simulink model "wlan_phy_rx_pmd"  (Simulink 7.8) (continued)
== system wlan_phy_rx_pmd/EDK Processor == (continued)
      | signal fifo_bank_out_i      : std_logic_vector(C_S_AXI_DATA_WIDTH-1 downto 0);
      | signal shmem_bank_out_i     : std_logic_vector(C_S_AXI_DATA_WIDTH-1 downto 0);
      |     
      | -- 'From Register'
      | -- 'RX_PKT_RSSI_CD'
      | signal sm_RX_PKT_RSSI_CD_dout_i  : std_logic_vector(C_S_AXI_DATA_WIDTH-1 downto 0);
      | -- 'RX_PKT_AGC_GAINS'
      | signal sm_RX_PKT_AGC_GAINS_dout_i  : std_logic_vector(C_S_AXI_DATA_WIDTH-1 downto 0);
      | -- 'RX_PKT_RSSI_AB'
      | signal sm_RX_PKT_RSSI_AB_dout_i  : std_logic_vector(C_S_AXI_DATA_WIDTH-1 downto 0);
      | -- 'CFO_EST_TIME_DOMAIN'
      | signal sm_CFO_EST_TIME_DOMAIN_dout_i  : std_logic_vector(C_S_AXI_DATA_WIDTH-1 downto 0);
      | -- 'Status'
      | signal sm_Status_dout_i  : std_logic_vector(C_S_AXI_DATA_WIDTH-1 downto 0);
      | -- 'To Register'
      | -- 'DEBUG_GPIO'
      | signal sm_DEBUG_GPIO_din_i   : std_logic_vector(C_S_AXI_DATA_WIDTH-1 downto 0);
      | signal sm_DEBUG_GPIO_en_i    : std_logic;
      | signal sm_DEBUG_GPIO_dout_i  : std_logic_vector(C_S_AXI_DATA_WIDTH-1 downto 0);
      | -- 'RSSI_THRESH'
      | signal sm_RSSI_THRESH_din_i   : std_logic_vector(C_S_AXI_DATA_WIDTH-1 downto 0);
      | signal sm_RSSI_THRESH_en_i    : std_logic;
      | signal sm_RSSI_THRESH_dout_i  : std_logic_vector(C_S_AXI_DATA_WIDTH-1 downto 0);
      | -- 'CONFIG'
      | signal sm_CONFIG_din_i   : std_logic_vector(C_S_AXI_DATA_WIDTH-1 downto 0);
      | signal sm_CONFIG_en_i    : std_logic;
      | signal sm_CONFIG_dout_i  : std_logic_vector(C_S_AXI_DATA_WIDTH-1 downto 0);
      | -- 'DSSS_RX_CONFIG'
      | signal sm_DSSS_RX_CONFIG_din_i   : std_logic_vector(C_S_AXI_DATA_WIDTH-1 downto 0);
      | signal sm_DSSS_RX_CONFIG_en_i    : std_logic;
      | signal sm_DSSS_RX_CONFIG_dout_i  : std_logic_vector(C_S_AXI_DATA_WIDTH-1 downto 0);
      | -- 'PKT_BUF_SEL'
      | signal sm_PKT_BUF_SEL_din_i   : std_logic_vector(C_S_AXI_DATA_WIDTH-1 downto 0);
      | signal sm_PKT_BUF_SEL_en_i    : std_logic;
      | signal sm_PKT_BUF_SEL_dout_i  : std_logic_vector(C_S_AXI_DATA_WIDTH-1 downto 0);
      | -- 'PKTDET_AUTOCORR_CONFIG'
      | signal sm_PKTDET_AUTOCORR_CONFIG_din_i   : std_logic_vector(C_S_AXI_DATA_WIDTH-1 downto 0);
      | signal sm_PKTDET_AUTOCORR_CONFIG_en_i    : std_logic;
      | signal sm_PKTDET_AUTOCORR_CONFIG_dout_i  : std_logic_vector(C_S_AXI_DATA_WIDTH-1 downto 0);
      | -- 'FEC_CONFIG'
      | signal sm_FEC_CONFIG_din_i   : std_logic_vector(C_S_AXI_DATA_WIDTH-1 downto 0);
      | signal sm_FEC_CONFIG_en_i    : std_logic;
      | signal sm_FEC_CONFIG_dout_i  : std_logic_vector(C_S_AXI_DATA_WIDTH-1 downto 0);
      | -- 'Control'
      | signal sm_Control_din_i   : std_logic_vector(C_S_AXI_DATA_WIDTH-1 downto 0);
      | signal sm_Control_en_i    : std_logic;
      | signal sm_Control_dout_i  : std_logic_vector(C_S_AXI_DATA_WIDTH-1 downto 0);
      | -- 'CHAN_EST_SMOOTHING'
      | signal sm_CHAN_EST_SMOOTHING_din_i   : std_logic_vector(C_S_AXI_DATA_WIDTH-1 downto 0);
      | signal sm_CHAN_EST_SMOOTHING_en_i    : std_logic;
      | signal sm_CHAN_EST_SMOOTHING_dout_i  : std_logic_vector(C_S_AXI_DATA_WIDTH-1 downto 0);
      | -- 'LTS_Corr_Thresh'
      | signal sm_LTS_Corr_Thresh_din_i   : std_logic_vector(C_S_AXI_DATA_WIDTH-1 downto 0);
      | signal sm_LTS_Corr_Thresh_en_i    : std_logic;
      | signal sm_LTS_Corr_Thresh_dout_i  : std_logic_vector(C_S_AXI_DATA_WIDTH-1 downto 0);
      | -- 'PKTDET_DSSS_CONFIG'
      | signal sm_PKTDET_DSSS_CONFIG_din_i   : std_logic_vector(C_S_AXI_DATA_WIDTH-1 downto 0);
      | signal sm_PKTDET_DSSS_CONFIG_en_i    : std_logic;
      | signal sm_PKTDET_DSSS_CONFIG_dout_i  : std_logic_vector(C_S_AXI_DATA_WIDTH-1 downto 0);
      | -- 'LTS_Corr_Config'
      | signal sm_LTS_Corr_Config_din_i   : std_logic_vector(C_S_AXI_DATA_WIDTH-1 downto 0);
      | signal sm_LTS_Corr_Config_en_i    : std_logic;
      | signal sm_LTS_Corr_Config_dout_i  : std_logic_vector(C_S_AXI_DATA_WIDTH-1 downto 0);
      | -- 'PHY_CCA_CONFIG'
      | signal sm_PHY_CCA_CONFIG_din_i   : std_logic_vector(C_S_AXI_DATA_WIDTH-1 downto 0);
      | signal sm_PHY_CCA_CONFIG_en_i    : std_logic;
      | signal sm_PHY_CCA_CONFIG_dout_i  : std_logic_vector(C_S_AXI_DATA_WIDTH-1 downto 0);
      | -- 'PKTBUF_MAX_WRITE_ADDR'
      | signal sm_PKTBUF_MAX_WRITE_ADDR_din_i   : std_logic_vector(C_S_AXI_DATA_WIDTH-1 downto 0);
      | signal sm_PKTBUF_MAX_WRITE_ADDR_en_i    : std_logic;
      | signal sm_PKTBUF_MAX_WRITE_ADDR_dout_i  : std_logic_vector(C_S_AXI_DATA_WIDTH-1 downto 0);
      | -- 'FFT_Config'
      | signal sm_FFT_Config_din_i   : std_logic_vector(C_S_AXI_DATA_WIDTH-1 downto 0);
      | signal sm_FFT_Config_en_i    : std_logic;
      | signal sm_FFT_Config_dout_i  : std_logic_vector(C_S_AXI_DATA_WIDTH-1 downto 0);
      | -- 'PKTDET_RSSI_CONFIG'
      | signal sm_PKTDET_RSSI_CONFIG_din_i   : std_logic_vector(C_S_AXI_DATA_WIDTH-1 downto 0);
      | signal sm_PKTDET_RSSI_CONFIG_en_i    : std_logic;
      | signal sm_PKTDET_RSSI_CONFIG_dout_i  : std_logic_vector(C_S_AXI_DATA_WIDTH-1 downto 0);
      | -- 'From FIFO'
      | -- 'To FIFO'
      | -- 'Shared Memory'
      | 
      | type t_read_state is (IDLE, READ_PREP, READ_DATA);
      | signal read_state : t_read_state;
      | 
      | type t_write_state is (IDLE, WRITE_DATA, WRITE_RESPONSE);
      | signal write_state : t_write_state;
      | 
      | type t_memmap_state is (READ, WRITE);
      | signal memmap_state : t_memmap_state;
      | 
      | constant C_READ_PREP_DELAY : std_logic_vector(1 downto 0) := "11";
      | 
      | signal read_prep_counter : std_logic_vector(1 downto 0);
      | signal read_addr_counter : std_logic_vector(C_S_AXI_ARLEN_WIDTH-1 downto 0);
      | signal read_data_counter : std_logic_vector(C_S_AXI_ARLEN_WIDTH-1 downto 0);
      | 
      | -- enable of shared BRAMs
      | signal s_shram_en : std_logic;
      | 
      | signal write_addr_valid : std_logic;
      | signal write_ready : std_logic;
      | 
      | -- 're' of From/To FIFOs
      | signal s_fifo_re : std_logic;
      | -- 'we' of To FIFOs
      | signal s_fifo_we : std_logic;
      | 
      | begin
      | 
      | -- enable for 'Shared Memory' blocks
      | 
      | -- conversion to match with the data bus width
      | -- 'From Register'
      | -- 'RX_PKT_RSSI_CD'
      | gen_sm_RX_PKT_RSSI_CD_dout_i: if (32 < C_S_AXI_DATA_WIDTH) generate
      |     sm_RX_PKT_RSSI_CD_dout_i(C_S_AXI_DATA_WIDTH-1 downto 32) <= (others => '0');
      | end generate gen_sm_RX_PKT_RSSI_CD_dout_i;
      | sm_RX_PKT_RSSI_CD_dout_i(32-1 downto 0) <= sm_RX_PKT_RSSI_CD_dout;
      | -- 'RX_PKT_AGC_GAINS'
      | gen_sm_RX_PKT_AGC_GAINS_dout_i: if (32 < C_S_AXI_DATA_WIDTH) generate
      |     sm_RX_PKT_AGC_GAINS_dout_i(C_S_AXI_DATA_WIDTH-1 downto 32) <= (others => '0');
      | end generate gen_sm_RX_PKT_AGC_GAINS_dout_i;
      | sm_RX_PKT_AGC_GAINS_dout_i(32-1 downto 0) <= sm_RX_PKT_AGC_GAINS_dout;
      | -- 'RX_PKT_RSSI_AB'
      | gen_sm_RX_PKT_RSSI_AB_dout_i: if (32 < C_S_AXI_DATA_WIDTH) generate
      |     sm_RX_PKT_RSSI_AB_dout_i(C_S_AXI_DATA_WIDTH-1 downto 32) <= (others => '0');
      | end generate gen_sm_RX_PKT_RSSI_AB_dout_i;
      | sm_RX_PKT_RSSI_AB_dout_i(32-1 downto 0) <= sm_RX_PKT_RSSI_AB_dout;
      | -- 'CFO_EST_TIME_DOMAIN'
      | gen_sm_CFO_EST_TIME_DOMAIN_dout_i: if (32 < C_S_AXI_DATA_WIDTH) generate
      |     sm_CFO_EST_TIME_DOMAIN_dout_i(C_S_AXI_DATA_WIDTH-1 downto 32) <= (others => '0');
      | end generate gen_sm_CFO_EST_TIME_DOMAIN_dout_i;
      | sm_CFO_EST_TIME_DOMAIN_dout_i(32-1 downto 0) <= sm_CFO_EST_TIME_DOMAIN_dout;
      | -- 'Status'
      | gen_sm_Status_dout_i: if (32 < C_S_AXI_DATA_WIDTH) generate
      |     sm_Status_dout_i(C_S_AXI_DATA_WIDTH-1 downto 32) <= (others => '0');
      | end generate gen_sm_Status_dout_i;
      | sm_Status_dout_i(32-1 downto 0) <= sm_Status_dout;
      | -- 'To Register'
      | -- 'DEBUG_GPIO'
      | sm_DEBUG_GPIO_din     <= sm_DEBUG_GPIO_din_i(32-1 downto 0);
      | sm_DEBUG_GPIO_en      <= sm_DEBUG_GPIO_en_i;
      | gen_sm_DEBUG_GPIO_dout_i: if (32 < C_S_AXI_DATA_WIDTH) generate
      |     sm_DEBUG_GPIO_dout_i(C_S_AXI_DATA_WIDTH-1 downto 32) <= (others => '0');
      | end generate gen_sm_DEBUG_GPIO_dout_i;
      | sm_DEBUG_GPIO_dout_i(32-1 downto 0) <= sm_DEBUG_GPIO_dout;
      | -- 'RSSI_THRESH'
      | sm_RSSI_THRESH_din     <= sm_RSSI_THRESH_din_i(32-1 downto 0);
      | sm_RSSI_THRESH_en      <= sm_RSSI_THRESH_en_i;
      | gen_sm_RSSI_THRESH_dout_i: if (32 < C_S_AXI_DATA_WIDTH) generate
      |     sm_RSSI_THRESH_dout_i(C_S_AXI_DATA_WIDTH-1 downto 32) <= (others => '0');
      | end generate gen_sm_RSSI_THRESH_dout_i;
      | sm_RSSI_THRESH_dout_i(32-1 downto 0) <= sm_RSSI_THRESH_dout;
      | -- 'CONFIG'
      | sm_CONFIG_din     <= sm_CONFIG_din_i(32-1 downto 0);
      | sm_CONFIG_en      <= sm_CONFIG_en_i;
      | gen_sm_CONFIG_dout_i: if (32 < C_S_AXI_DATA_WIDTH) generate
      |     sm_CONFIG_dout_i(C_S_AXI_DATA_WIDTH-1 downto 32) <= (others => '0');
      | end generate gen_sm_CONFIG_dout_i;
      | sm_CONFIG_dout_i(32-1 downto 0) <= sm_CONFIG_dout;
      | -- 'DSSS_RX_CONFIG'
      | sm_DSSS_RX_CONFIG_din     <= sm_DSSS_RX_CONFIG_din_i(32-1 downto 0);
      | sm_DSSS_RX_CONFIG_en      <= sm_DSSS_RX_CONFIG_en_i;
      | gen_sm_DSSS_RX_CONFIG_dout_i: if (32 < C_S_AXI_DATA_WIDTH) generate
      |     sm_DSSS_RX_CONFIG_dout_i(C_S_AXI_DATA_WIDTH-1 downto 32) <= (others => '0');
      | end generate gen_sm_DSSS_RX_CONFIG_dout_i;
      | sm_DSSS_RX_CONFIG_dout_i(32-1 downto 0) <= sm_DSSS_RX_CONFIG_dout;
      | -- 'PKT_BUF_SEL'
      | sm_PKT_BUF_SEL_din     <= sm_PKT_BUF_SEL_din_i(32-1 downto 0);
      | sm_PKT_BUF_SEL_en      <= sm_PKT_BUF_SEL_en_i;
      | gen_sm_PKT_BUF_SEL_dout_i: if (32 < C_S_AXI_DATA_WIDTH) generate
      |     sm_PKT_BUF_SEL_dout_i(C_S_AXI_DATA_WIDTH-1 downto 32) <= (others => '0');
      | end generate gen_sm_PKT_BUF_SEL_dout_i;
      | sm_PKT_BUF_SEL_dout_i(32-1 downto 0) <= sm_PKT_BUF_SEL_dout;
      | -- 'PKTDET_AUTOCORR_CONFIG'
      | sm_PKTDET_AUTOCORR_CONFIG_din     <= sm_PKTDET_AUTOCORR_CONFIG_din_i(32-1 downto 0);
      | sm_PKTDET_AUTOCORR_CONFIG_en      <= sm_PKTDET_AUTOCORR_CONFIG_en_i;
      | gen_sm_PKTDET_AUTOCORR_CONFIG_dout_i: if (32 < C_S_AXI_DATA_WIDTH) generate
      |     sm_PKTDET_AUTOCORR_CONFIG_dout_i(C_S_AXI_DATA_WIDTH-1 downto 32) <= (others => '0');
      | end generate gen_sm_PKTDET_AUTOCORR_CONFIG_dout_i;
      | sm_PKTDET_AUTOCORR_CONFIG_dout_i(32-1 downto 0) <= sm_PKTDET_AUTOCORR_CONFIG_dout;
      | -- 'FEC_CONFIG'
      | sm_FEC_CONFIG_din     <= sm_FEC_CONFIG_din_i(32-1 downto 0);
      | sm_FEC_CONFIG_en      <= sm_FEC_CONFIG_en_i;
      | gen_sm_FEC_CONFIG_dout_i: if (32 < C_S_AXI_DATA_WIDTH) generate
      |     sm_FEC_CONFIG_dout_i(C_S_AXI_DATA_WIDTH-1 downto 32) <= (others => '0');
      | end generate gen_sm_FEC_CONFIG_dout_i;
      | sm_FEC_CONFIG_dout_i(32-1 downto 0) <= sm_FEC_CONFIG_dout;
      | -- 'Control'
      | sm_Control_din     <= sm_Control_din_i(32-1 downto 0);
      | sm_Control_en      <= sm_Control_en_i;
      | gen_sm_Control_dout_i: if (32 < C_S_AXI_DATA_WIDTH) generate
      |     sm_Control_dout_i(C_S_AXI_DATA_WIDTH-1 downto 32) <= (others => '0');
      | end generate gen_sm_Control_dout_i;
      | sm_Control_dout_i(32-1 downto 0) <= sm_Control_dout;
      | -- 'CHAN_EST_SMOOTHING'
      | sm_CHAN_EST_SMOOTHING_din     <= sm_CHAN_EST_SMOOTHING_din_i(32-1 downto 0);
      | sm_CHAN_EST_SMOOTHING_en      <= sm_CHAN_EST_SMOOTHING_en_i;
      | gen_sm_CHAN_EST_SMOOTHING_dout_i: if (32 < C_S_AXI_DATA_WIDTH) generate
      |     sm_CHAN_EST_SMOOTHING_dout_i(C_S_AXI_DATA_WIDTH-1 downto 32) <= (others => '0');
      | end generate gen_sm_CHAN_EST_SMOOTHING_dout_i;
      | sm_CHAN_EST_SMOOTHING_dout_i(32-1 downto 0) <= sm_CHAN_EST_SMOOTHING_dout;
      | -- 'LTS_Corr_Thresh'
      | sm_LTS_Corr_Thresh_din     <= sm_LTS_Corr_Thresh_din_i(32-1 downto 0);
      | sm_LTS_Corr_Thresh_en      <= sm_LTS_Corr_Thresh_en_i;
      | gen_sm_LTS_Corr_Thresh_dout_i: if (32 < C_S_AXI_DATA_WIDTH) generate
      |     sm_LTS_Corr_Thresh_dout_i(C_S_AXI_DATA_WIDTH-1 downto 32) <= (others => '0');
      | end generate gen_sm_LTS_Corr_Thresh_dout_i;
      | sm_LTS_Corr_Thresh_dout_i(32-1 downto 0) <= sm_LTS_Corr_Thresh_dout;
      | -- 'PKTDET_DSSS_CONFIG'
      | sm_PKTDET_DSSS_CONFIG_din     <= sm_PKTDET_DSSS_CONFIG_din_i(32-1 downto 0);
      | sm_PKTDET_DSSS_CONFIG_en      <= sm_PKTDET_DSSS_CONFIG_en_i;
      | gen_sm_PKTDET_DSSS_CONFIG_dout_i: if (32 < C_S_AXI_DATA_WIDTH) generate
      |     sm_PKTDET_DSSS_CONFIG_dout_i(C_S_AXI_DATA_WIDTH-1 downto 32) <= (others => '0');
      | end generate gen_sm_PKTDET_DSSS_CONFIG_dout_i;
      | sm_PKTDET_DSSS_CONFIG_dout_i(32-1 downto 0) <= sm_PKTDET_DSSS_CONFIG_dout;
      | -- 'LTS_Corr_Config'
      | sm_LTS_Corr_Config_din     <= sm_LTS_Corr_Config_din_i(32-1 downto 0);
      | sm_LTS_Corr_Config_en      <= sm_LTS_Corr_Config_en_i;
      | gen_sm_LTS_Corr_Config_dout_i: if (32 < C_S_AXI_DATA_WIDTH) generate
      |     sm_LTS_Corr_Config_dout_i(C_S_AXI_DATA_WIDTH-1 downto 32) <= (others => '0');
      | end generate gen_sm_LTS_Corr_Config_dout_i;
      | sm_LTS_Corr_Config_dout_i(32-1 downto 0) <= sm_LTS_Corr_Config_dout;
      | -- 'PHY_CCA_CONFIG'
      | sm_PHY_CCA_CONFIG_din     <= sm_PHY_CCA_CONFIG_din_i(32-1 downto 0);
      | sm_PHY_CCA_CONFIG_en      <= sm_PHY_CCA_CONFIG_en_i;
      | gen_sm_PHY_CCA_CONFIG_dout_i: if (32 < C_S_AXI_DATA_WIDTH) generate
      |     sm_PHY_CCA_CONFIG_dout_i(C_S_AXI_DATA_WIDTH-1 downto 32) <= (others => '0');
      | end generate gen_sm_PHY_CCA_CONFIG_dout_i;
      | sm_PHY_CCA_CONFIG_dout_i(32-1 downto 0) <= sm_PHY_CCA_CONFIG_dout;
      | -- 'PKTBUF_MAX_WRITE_ADDR'
      | sm_PKTBUF_MAX_WRITE_ADDR_din     <= sm_PKTBUF_MAX_WRITE_ADDR_din_i(32-1 downto 0);
      | sm_PKTBUF_MAX_WRITE_ADDR_en      <= sm_PKTBUF_MAX_WRITE_ADDR_en_i;
      | gen_sm_PKTBUF_MAX_WRITE_ADDR_dout_i: if (32 < C_S_AXI_DATA_WIDTH) generate
      |     sm_PKTBUF_MAX_WRITE_ADDR_dout_i(C_S_AXI_DATA_WIDTH-1 downto 32) <= (others => '0');
      | end generate gen_sm_PKTBUF_MAX_WRITE_ADDR_dout_i;
      | sm_PKTBUF_MAX_WRITE_ADDR_dout_i(32-1 downto 0) <= sm_PKTBUF_MAX_WRITE_ADDR_dout;
      | -- 'FFT_Config'
      | sm_FFT_Config_din     <= sm_FFT_Config_din_i(32-1 downto 0);
      | sm_FFT_Config_en      <= sm_FFT_Config_en_i;
      | gen_sm_FFT_Config_dout_i: if (32 < C_S_AXI_DATA_WIDTH) generate
      |     sm_FFT_Config_dout_i(C_S_AXI_DATA_WIDTH-1 downto 32) <= (others => '0');
      | end generate gen_sm_FFT_Config_dout_i;
      | sm_FFT_Config_dout_i(32-1 downto 0) <= sm_FFT_Config_dout;
      | -- 'PKTDET_RSSI_CONFIG'
      | sm_PKTDET_RSSI_CONFIG_din     <= sm_PKTDET_RSSI_CONFIG_din_i(32-1 downto 0);
      | sm_PKTDET_RSSI_CONFIG_en      <= sm_PKTDET_RSSI_CONFIG_en_i;
      | gen_sm_PKTDET_RSSI_CONFIG_dout_i: if (32 < C_S_AXI_DATA_WIDTH) generate
      |     sm_PKTDET_RSSI_CONFIG_dout_i(C_S_AXI_DATA_WIDTH-1 downto 32) <= (others => '0');
      | end generate gen_sm_PKTDET_RSSI_CONFIG_dout_i;
      | sm_PKTDET_RSSI_CONFIG_dout_i(32-1 downto 0) <= sm_PKTDET_RSSI_CONFIG_dout;
      | -- 'From FIFO'
      | -- 'To FIFO'
      | -- 'Shared Memory'
      | 
      | ReadWriteSelect: process(memmap_state) is begin
      |     if (memmap_state = READ) then
      |     else
      |     end if;
      | end process ReadWriteSelect;
      | 
      | -----------------------------------------------------------------------------
      | -- address for 'Shared Memory'
      | -----------------------------------------------------------------------------
      | SharedMemory_Addr_ResetN : process(AXI_AClk) is begin
      |     if (AXI_AClk'event and AXI_AClk = '1') then
      |         if (AXI_AResetN = '0') then
      |             memmap_state <= READ;
      |         else
      |             if (S_AXI_AWVALID = '1') then
      |                 -- write operation
      |                 memmap_state <= WRITE;
      |             elsif (S_AXI_ARVALID = '1') then
      |                 -- read operation
      |                 memmap_state <= READ;
      |             end if;
      |         end if;
      |     end if;
      | end process SharedMemory_Addr_ResetN;
      | 
      | -----------------------------------------------------------------------------
      | -- WRITE Command Control
      | -----------------------------------------------------------------------------
      | S_AXI_BID     <= S_AXI_BID_i;
      | S_AXI_BVALID  <= S_AXI_BVALID_i;
      | S_AXI_WREADY  <= S_AXI_WREADY_i;
      | -- No error checking
      | S_AXI_BRESP  <= (others=>'0');
      | 
      | PROC_AWREADY_ACK: process(read_state, write_state, S_AXI_ARVALID, S_AXI_AWVALID) is begin
      |     if (write_state = IDLE and S_AXI_AWVALID = '1' and read_state = IDLE) then
      |         S_AXI_AWREADY <= S_AXI_AWVALID;
      |     else
      |         S_AXI_AWREADY <= '0';
      |     end if;
      | end process PROC_AWREADY_ACK;
      | 
      | Cmd_Decode_Write: process(AXI_AClk) is begin
      |     if (AXI_AClk'event and AXI_AClk = '1') then
      |         if (AXI_AResetN = '0') then
      |             write_addr_valid    <= '0';
      |             write_ready         <= '0';
      |             s_fifo_we           <= '0';
      |             S_AXI_BVALID_i      <= '0';
      |             S_AXI_BID_i         <= (others => '0');
      |             write_bank_addr_i   <= (others => '0');
      |             write_linear_addr_i <= (others => '0');
      |         else
      |             if (write_state = IDLE) then
      |                 if (S_AXI_AWVALID = '1' and read_state = IDLE) then
      |                     -- reflect awid
      |                     S_AXI_BID_i <= S_AXI_AWID;
      | 
      |                     -- latch bank and linear addresses
      |                     write_bank_addr_i   <= S_AXI_AWADDR(C_S_AXI_TOTAL_ADDR_LEN-1 downto C_S_AXI_LINEAR_ADDR_LEN+2);
      |                     write_linear_addr_i <= S_AXI_AWADDR(C_S_AXI_LINEAR_ADDR_LEN+1 downto 2);
      |                     write_addr_valid <= '1';
      |                     s_fifo_we <= '1';
      | 
      |                     -- write state transition
      |                     write_state <= WRITE_DATA;
      |                 end if;
      |             elsif (write_state = WRITE_DATA) then
      |                 write_ready <= '1';
      |                 s_fifo_we <= '0';
      |                 write_addr_valid <= S_AXI_WVALID;
      |                 
      |                 if (S_AXI_WVALID = '1' and write_ready = '1') then
      |                     write_linear_addr_i <= Std_Logic_Vector(unsigned(write_linear_addr_i) + 1);
      |                 end if;
      | 
      |                 if (S_AXI_WLAST = '1' and write_ready = '1') then
      |                     -- start responding through B channel upon the last write data sample
      |                     S_AXI_BVALID_i <= '1';
      |                     -- write data is over
      |                     write_addr_valid <= '0';
      |                     write_ready <= '0';
      |                     -- write state transition
      |                     write_state <= WRITE_RESPONSE;
      |                 end if;
      |             elsif (write_state = WRITE_RESPONSE) then
      | 
      |                 if (S_AXI_BREADY = '1') then
      |                     -- write respond is over
      |                     S_AXI_BVALID_i <= '0';
      |                     S_AXI_BID_i <= (others => '0');
      | 
      |                     -- write state transition
      |                     write_state <= IDLE;
      |                 end if;
      |             end if;
      |         end if;
      |     end if;
      | end process Cmd_Decode_Write;
      | 
      | Write_Linear_Addr_Decode : process(AXI_AClk) is 
      | 
      | begin
      |     if (AXI_AClk'event and AXI_AClk = '1') then
      |         if (AXI_AResetN = '0') then
      |             -- 'To Register'
      |             -- DEBUG_GPIO din/en
      |             sm_DEBUG_GPIO_din_i <= (others => '0');
      |             sm_DEBUG_GPIO_en_i <= '0';
      |             -- RSSI_THRESH din/en
      |             sm_RSSI_THRESH_din_i <= (others => '0');
      |             sm_RSSI_THRESH_en_i <= '0';
      |             -- CONFIG din/en
      |             sm_CONFIG_din_i <= (others => '0');
      |             sm_CONFIG_en_i <= '0';
      |             -- DSSS_RX_CONFIG din/en
      |             sm_DSSS_RX_CONFIG_din_i <= (others => '0');
      |             sm_DSSS_RX_CONFIG_en_i <= '0';
      |             -- PKT_BUF_SEL din/en
      |             sm_PKT_BUF_SEL_din_i <= (others => '0');
      |             sm_PKT_BUF_SEL_en_i <= '0';
      |             -- PKTDET_AUTOCORR_CONFIG din/en
      |             sm_PKTDET_AUTOCORR_CONFIG_din_i <= (others => '0');
      |             sm_PKTDET_AUTOCORR_CONFIG_en_i <= '0';
      |             -- FEC_CONFIG din/en
      |             sm_FEC_CONFIG_din_i <= (others => '0');
      |             sm_FEC_CONFIG_en_i <= '0';
      |             -- Control din/en
      |             sm_Control_din_i <= (others => '0');
      |             sm_Control_en_i <= '0';
      |             -- CHAN_EST_SMOOTHING din/en
      |             sm_CHAN_EST_SMOOTHING_din_i <= (others => '0');
      |             sm_CHAN_EST_SMOOTHING_en_i <= '0';
      |             -- LTS_Corr_Thresh din/en
      |             sm_LTS_Corr_Thresh_din_i <= (others => '0');
      |             sm_LTS_Corr_Thresh_en_i <= '0';
      |             -- PKTDET_DSSS_CONFIG din/en
      |             sm_PKTDET_DSSS_CONFIG_din_i <= (others => '0');
      |             sm_PKTDET_DSSS_CONFIG_en_i <= '0';
      |             -- LTS_Corr_Config din/en
      |             sm_LTS_Corr_Config_din_i <= (others => '0');
      |             sm_LTS_Corr_Config_en_i <= '0';
      |             -- PHY_CCA_CONFIG din/en
      |             sm_PHY_CCA_CONFIG_din_i <= (others => '0');
      |             sm_PHY_CCA_CONFIG_en_i <= '0';
      |             -- PKTBUF_MAX_WRITE_ADDR din/en
      |             sm_PKTBUF_MAX_WRITE_ADDR_din_i <= (others => '0');
      |             sm_PKTBUF_MAX_WRITE_ADDR_en_i <= '0';
      |             -- FFT_Config din/en
      |             sm_FFT_Config_din_i <= (others => '0');
      |             sm_FFT_Config_en_i <= '0';
      |             -- PKTDET_RSSI_CONFIG din/en
      |             sm_PKTDET_RSSI_CONFIG_din_i <= (others => '0');
      |             sm_PKTDET_RSSI_CONFIG_en_i <= '0';
      |             -- 'To FIFO'
      |             -- 'Shared Memory'
      |         else
      |             -- default assignments
      | 
      |             -- 'To Register'
      |             if (unsigned(write_bank_addr_i) = 2) then
      |                 if (unsigned(write_linear_addr_i) = 0) then
      |                     -- DEBUG_GPIO din/en
      |                     sm_DEBUG_GPIO_din_i <= S_AXI_WDATA;
      |                     sm_DEBUG_GPIO_en_i  <= write_addr_valid;
      |                 elsif (unsigned(write_linear_addr_i) = 1) then
      |                     -- RSSI_THRESH din/en
      |                     sm_RSSI_THRESH_din_i <= S_AXI_WDATA;
      |                     sm_RSSI_THRESH_en_i  <= write_addr_valid;
      |                 elsif (unsigned(write_linear_addr_i) = 2) then
      |                     -- CONFIG din/en
      |                     sm_CONFIG_din_i <= S_AXI_WDATA;
      |                     sm_CONFIG_en_i  <= write_addr_valid;
      |                 elsif (unsigned(write_linear_addr_i) = 3) then
      |                     -- DSSS_RX_CONFIG din/en
      |                     sm_DSSS_RX_CONFIG_din_i <= S_AXI_WDATA;
      |                     sm_DSSS_RX_CONFIG_en_i  <= write_addr_valid;
      |                 elsif (unsigned(write_linear_addr_i) = 4) then
      |                     -- PKT_BUF_SEL din/en
      |                     sm_PKT_BUF_SEL_din_i <= S_AXI_WDATA;
      |                     sm_PKT_BUF_SEL_en_i  <= write_addr_valid;
      |                 elsif (unsigned(write_linear_addr_i) = 5) then
      |                     -- PKTDET_AUTOCORR_CONFIG din/en
      |                     sm_PKTDET_AUTOCORR_CONFIG_din_i <= S_AXI_WDATA;
      |                     sm_PKTDET_AUTOCORR_CONFIG_en_i  <= write_addr_valid;
      |                 elsif (unsigned(write_linear_addr_i) = 6) then
      |                     -- FEC_CONFIG din/en
      |                     sm_FEC_CONFIG_din_i <= S_AXI_WDATA;
      |                     sm_FEC_CONFIG_en_i  <= write_addr_valid;
      |                 elsif (unsigned(write_linear_addr_i) = 7) then
      |                     -- Control din/en
      |                     sm_Control_din_i <= S_AXI_WDATA;
      |                     sm_Control_en_i  <= write_addr_valid;
      |                 elsif (unsigned(write_linear_addr_i) = 8) then
      |                     -- CHAN_EST_SMOOTHING din/en
      |                     sm_CHAN_EST_SMOOTHING_din_i <= S_AXI_WDATA;
      |                     sm_CHAN_EST_SMOOTHING_en_i  <= write_addr_valid;
      |                 elsif (unsigned(write_linear_addr_i) = 9) then
      |                     -- LTS_Corr_Thresh din/en
      |                     sm_LTS_Corr_Thresh_din_i <= S_AXI_WDATA;
      |                     sm_LTS_Corr_Thresh_en_i  <= write_addr_valid;
      |                 elsif (unsigned(write_linear_addr_i) = 10) then
      |                     -- PKTDET_DSSS_CONFIG din/en
      |                     sm_PKTDET_DSSS_CONFIG_din_i <= S_AXI_WDATA;
      |                     sm_PKTDET_DSSS_CONFIG_en_i  <= write_addr_valid;
      |                 elsif (unsigned(write_linear_addr_i) = 11) then
      |                     -- LTS_Corr_Config din/en
      |                     sm_LTS_Corr_Config_din_i <= S_AXI_WDATA;
      |                     sm_LTS_Corr_Config_en_i  <= write_addr_valid;
      |                 elsif (unsigned(write_linear_addr_i) = 12) then
      |                     -- PHY_CCA_CONFIG din/en
      |                     sm_PHY_CCA_CONFIG_din_i <= S_AXI_WDATA;
      |                     sm_PHY_CCA_CONFIG_en_i  <= write_addr_valid;
      |                 elsif (unsigned(write_linear_addr_i) = 13) then
      |                     -- PKTBUF_MAX_WRITE_ADDR din/en
      |                     sm_PKTBUF_MAX_WRITE_ADDR_din_i <= S_AXI_WDATA;
      |                     sm_PKTBUF_MAX_WRITE_ADDR_en_i  <= write_addr_valid;
      |                 elsif (unsigned(write_linear_addr_i) = 14) then
      |                     -- FFT_Config din/en
      |                     sm_FFT_Config_din_i <= S_AXI_WDATA;
      |                     sm_FFT_Config_en_i  <= write_addr_valid;
      |                 elsif (unsigned(write_linear_addr_i) = 15) then
      |                     -- PKTDET_RSSI_CONFIG din/en
      |                     sm_PKTDET_RSSI_CONFIG_din_i <= S_AXI_WDATA;
      |                     sm_PKTDET_RSSI_CONFIG_en_i  <= write_addr_valid;
      |                 end if;
      |             end if;        
      |         
      |         
      |         end if;
      |     end if;
      | end process Write_Linear_Addr_Decode;
      |  
      | -----------------------------------------------------------------------------
      | -- READ Control
      | -----------------------------------------------------------------------------
      | 
      | S_AXI_RDATA  <= S_AXI_RDATA_i;
      | S_AXI_RVALID  <= S_AXI_RVALID_i;
      | S_AXI_RLAST   <= S_AXI_RLAST_i;
      | S_AXI_RID     <= S_AXI_RID_i;
      | -- TODO: no error checking
      | S_AXI_RRESP <= (others=>'0');
      | 
      | PROC_ARREADY_ACK: process(read_state, S_AXI_ARVALID, write_state, S_AXI_AWVALID) is begin
      |     -- Note: WRITE has higher priority than READ
      |     if (read_state = IDLE and S_AXI_ARVALID = '1' and write_state = IDLE and S_AXI_AWVALID /= '1') then
      |         S_AXI_ARREADY <= S_AXI_ARVALID;
      |     else
      |         S_AXI_ARREADY <= '0';
      |     end if;
      | end process PROC_ARREADY_ACK;
      | 
      | S_AXI_WREADY_i <= write_ready;
      | 
      | Process_Sideband: process(write_state, read_state) is begin
      |     if (read_state = READ_PREP) then
      |         s_shram_en <= '1';
      |     elsif (read_state = READ_DATA) then
      |         s_shram_en <= S_AXI_RREADY;
      |     elsif (write_state = WRITE_DATA) then
      |         s_shram_en <= S_AXI_WVALID;
      |     else
      |         s_shram_en <= '0';
      |     end if;
      | end process Process_Sideband;
      | 
      | Cmd_Decode_Read: process(AXI_AClk) is begin
      |     if (AXI_AClk'event and AXI_AClk = '1') then
      |         if (AXI_AResetN = '0') then
      |             S_AXI_RVALID_i <= '0';
      |             read_bank_addr_i    <= (others => '0');
      |             read_linear_addr_i  <= (others => '0');
      |             S_AXI_ARLEN_i       <= (others => '0');
      |             S_AXI_RLAST_i       <= '0';
      |             S_AXI_RID_i         <= (others => '0');
      |             read_state          <= IDLE;
      |             read_prep_counter   <= (others => '0');
      |             read_addr_counter   <= (others => '0');
      |             read_data_counter   <= (others => '0');
      |         else
      |             -- default assignments
      |             s_fifo_re <= '0';
      | 
      |             if (read_state = IDLE) then
      |                 -- Note WRITE has higher priority than READ
      |                 if (S_AXI_ARVALID = '1' and write_state = IDLE and S_AXI_AWVALID /= '1') then
      |                     -- extract bank and linear addresses
      |                     read_bank_addr_i    <= S_AXI_ARADDR(C_S_AXI_TOTAL_ADDR_LEN-1 downto C_S_AXI_LINEAR_ADDR_LEN+2);
      |                     read_linear_addr_i  <= S_AXI_ARADDR(C_S_AXI_LINEAR_ADDR_LEN+1 downto 2);
      |                     s_fifo_re <= '1';
      | 
      |                     -- reflect arid
      |                     S_AXI_RID_i <= S_AXI_ARID;
      | 
      |                     -- load read liner address and data counter
      |                     read_addr_counter <= S_AXI_ARLEN;
      |                     read_data_counter <= S_AXI_ARLEN;
      | 
      |                     -- load read preparation counter
      |                     read_prep_counter <= C_READ_PREP_DELAY;
      |                     -- read state transition
      |                     read_state <= READ_PREP;
      |                 end if;
      |             elsif (read_state = READ_PREP) then
      |                 if (unsigned(read_prep_counter) = 0) then
      |                     if (unsigned(read_data_counter) = 0) then
      |                         -- tag the last data generated by the slave
      |                         S_AXI_RLAST_i <= '1';
      |                     end if;
      |                     -- valid data appears
      |                     S_AXI_RVALID_i <= '1';
      |                     -- read state transition
      |                     read_state <= READ_DATA;
      |                 else
      |                     -- decrease read preparation counter
      |                     read_prep_counter <= Std_Logic_Vector(unsigned(read_prep_counter) - 1);
      |                 end if;
      | 
      |                 if (unsigned(read_prep_counter) /= 3 and unsigned(read_addr_counter) /= 0) then
      |                     -- decrease address counter
      |                     read_addr_counter <= Std_Logic_Vector(unsigned(read_addr_counter) - 1);
      |                     -- increase linear address (no band crossing)
      |                     read_linear_addr_i <= Std_Logic_Vector(unsigned(read_linear_addr_i) + 1);
      |                 end if;
      |             elsif (read_state = READ_DATA) then
      |                 if (S_AXI_RREADY = '1') then
      |                     if (unsigned(read_data_counter) = 1) then
      |                         -- tag the last data generated by the slave
      |                         S_AXI_RLAST_i <= '1';
      |                     end if;
      | 
      |                     if (unsigned(read_data_counter) = 0) then
      |                         -- arid
      |                         S_AXI_RID_i <= (others => '0');
      |                         -- rlast
      |                         S_AXI_RLAST_i <= '0';
      |                         -- no more valid data
      |                         S_AXI_RVALID_i <= '0';
      |                         -- read state transition
      |                         read_state <= IDLE;
      |                     else
      |                         -- decrease read preparation counter
      |                         read_data_counter <= Std_Logic_Vector(unsigned(read_data_counter) - 1);
      | 
      |                         if (unsigned(read_addr_counter) /= 0) then
      |                             -- decrease address counter
      |                             read_addr_counter <= Std_Logic_Vector(unsigned(read_addr_counter) - 1);
      |                             -- increase linear address (no band crossing)
      |                             read_linear_addr_i <= Std_Logic_Vector(unsigned(read_linear_addr_i) + 1);
      |                         end if;
      |                     end if;
      |                 end if;
      |             end if;
      | 
      |         end if;
      |     end if;
      | end process Cmd_Decode_Read;
      | 
      | Read_Linear_Addr_Decode : process(AXI_AClk) is begin
      |     if (AXI_AClk'event and AXI_AClk = '1') then
      |         if (AXI_AResetN = '0') then
      |             reg_bank_out_i   <= (others => '0');
      |             fifo_bank_out_i  <= (others => '0');
      |             shmem_bank_out_i <= (others => '0');
      |             S_AXI_RDATA_i    <= (others => '0');
      |         else
      |             if (unsigned(read_bank_addr_i) = 2) then
      |                 -- 'From Register'
      |                 if (unsigned(read_linear_addr_i) = 16) then
      |                     -- 'RX_PKT_RSSI_CD' dout
      |                     reg_bank_out_i <= sm_RX_PKT_RSSI_CD_dout_i;
      |                 elsif (unsigned(read_linear_addr_i) = 17) then
      |                     -- 'RX_PKT_AGC_GAINS' dout
      |                     reg_bank_out_i <= sm_RX_PKT_AGC_GAINS_dout_i;
      |                 elsif (unsigned(read_linear_addr_i) = 18) then
      |                     -- 'RX_PKT_RSSI_AB' dout
      |                     reg_bank_out_i <= sm_RX_PKT_RSSI_AB_dout_i;
      |                 elsif (unsigned(read_linear_addr_i) = 19) then
      |                     -- 'CFO_EST_TIME_DOMAIN' dout
      |                     reg_bank_out_i <= sm_CFO_EST_TIME_DOMAIN_dout_i;
      |                 elsif (unsigned(read_linear_addr_i) = 20) then
      |                     -- 'Status' dout
      |                     reg_bank_out_i <= sm_Status_dout_i;
      |                 end if;
      |                 -- 'To Register' (with register readback)
      |                 if (unsigned(read_linear_addr_i) = 0) then
      |                     -- 'DEBUG_GPIO' dout
      |                     reg_bank_out_i <= sm_DEBUG_GPIO_dout_i;
      |                 elsif (unsigned(read_linear_addr_i) = 1) then
      |                     -- 'RSSI_THRESH' dout
      |                     reg_bank_out_i <= sm_RSSI_THRESH_dout_i;
      |                 elsif (unsigned(read_linear_addr_i) = 2) then
      |                     -- 'CONFIG' dout
      |                     reg_bank_out_i <= sm_CONFIG_dout_i;
      |                 elsif (unsigned(read_linear_addr_i) = 3) then
      |                     -- 'DSSS_RX_CONFIG' dout
      |                     reg_bank_out_i <= sm_DSSS_RX_CONFIG_dout_i;
      |                 elsif (unsigned(read_linear_addr_i) = 4) then
      |                     -- 'PKT_BUF_SEL' dout
      |                     reg_bank_out_i <= sm_PKT_BUF_SEL_dout_i;
      |                 elsif (unsigned(read_linear_addr_i) = 5) then
      |                     -- 'PKTDET_AUTOCORR_CONFIG' dout
      |                     reg_bank_out_i <= sm_PKTDET_AUTOCORR_CONFIG_dout_i;
      |                 elsif (unsigned(read_linear_addr_i) = 6) then
      |                     -- 'FEC_CONFIG' dout
      |                     reg_bank_out_i <= sm_FEC_CONFIG_dout_i;
      |                 elsif (unsigned(read_linear_addr_i) = 7) then
      |                     -- 'Control' dout
      |                     reg_bank_out_i <= sm_Control_dout_i;
      |                 elsif (unsigned(read_linear_addr_i) = 8) then
      |                     -- 'CHAN_EST_SMOOTHING' dout
      |                     reg_bank_out_i <= sm_CHAN_EST_SMOOTHING_dout_i;
      |                 elsif (unsigned(read_linear_addr_i) = 9) then
      |                     -- 'LTS_Corr_Thresh' dout
      |                     reg_bank_out_i <= sm_LTS_Corr_Thresh_dout_i;
      |                 elsif (unsigned(read_linear_addr_i) = 10) then
      |                     -- 'PKTDET_DSSS_CONFIG' dout
      |                     reg_bank_out_i <= sm_PKTDET_DSSS_CONFIG_dout_i;
      |                 elsif (unsigned(read_linear_addr_i) = 11) then
      |                     -- 'LTS_Corr_Config' dout
      |                     reg_bank_out_i <= sm_LTS_Corr_Config_dout_i;
      |                 elsif (unsigned(read_linear_addr_i) = 12) then
      |                     -- 'PHY_CCA_CONFIG' dout
      |                     reg_bank_out_i <= sm_PHY_CCA_CONFIG_dout_i;
      |                 elsif (unsigned(read_linear_addr_i) = 13) then
      |                     -- 'PKTBUF_MAX_WRITE_ADDR' dout
      |                     reg_bank_out_i <= sm_PKTBUF_MAX_WRITE_ADDR_dout_i;
      |                 elsif (unsigned(read_linear_addr_i) = 14) then
      |                     -- 'FFT_Config' dout
      |                     reg_bank_out_i <= sm_FFT_Config_dout_i;
      |                 elsif (unsigned(read_linear_addr_i) = 15) then
      |                     -- 'PKTDET_RSSI_CONFIG' dout
      |                     reg_bank_out_i <= sm_PKTDET_RSSI_CONFIG_dout_i;
      |                 end if;
      | 
      |                 S_AXI_RDATA_i <= reg_bank_out_i;
      |             elsif (unsigned(read_bank_addr_i) = 1) then
      |                 -- 'From FIFO'
      |                 -- 'To FIFO'
      | 
      |                 S_AXI_RDATA_i <= fifo_bank_out_i;
      |             elsif (unsigned(read_bank_addr_i) = 0 and s_shram_en = '1') then
      |                 -- 'Shared Memory'
      | 
      |                 S_AXI_RDATA_i <= shmem_bank_out_i;
      |             end if;
      |         end if;
      |     end if;
      | end process Read_Linear_Addr_Decode;
      | 
      | end architecture IMP;
      settings: infoedit=For use with EDK Processor block.
  wires:
  memmap/15 -> To Register1/2
  memmap/14 -> To Register1/1
  memmap/21 -> To Register4/2
  memmap/20 -> To Register4/1
  memmap/43 -> To Register15/2
  memmap/42 -> To Register15/1
  memmap/33 -> To Register10/2
  memmap/32 -> To Register10/1
  memmap/23 -> To Register5/2
  memmap/22 -> To Register5/1
  memmap/39 -> To Register13/2
  memmap/38 -> To Register13/1
  memmap/37 -> To Register12/2
  memmap/36 -> To Register12/1
  memmap/31 -> To Register9/2
  memmap/30 -> To Register9/1
  memmap/35 -> To Register11/2
  memmap/34 -> To Register11/1
  memmap/41 -> To Register14/2
  memmap/40 -> To Register14/1
  memmap/25 -> To Register6/2
  memmap/24 -> To Register6/1
  memmap/19 -> To Register3/2
  memmap/18 -> To Register3/1
  memmap/13 -> To Register/2
  memmap/12 -> To Register/1
  memmap/27 -> To Register7/2
  memmap/26 -> To Register7/1
  memmap/17 -> To Register2/2
  memmap/16 -> To Register2/1
  memmap/29 -> To Register8/2
  memmap/28 -> To Register8/1
  memmap/11 -> S_AXI_WREADY/1
  memmap/10 -> S_AXI_RVALID/1
  memmap/9 -> S_AXI_RRESP/1
  memmap/8 -> S_AXI_RLAST/1
  memmap/7 -> S_AXI_RID/1
  memmap/6 -> S_AXI_RDATA/1
  memmap/5 -> S_AXI_BVALID/1
  memmap/4 -> S_AXI_BRESP/1
  memmap/3 -> S_AXI_BID/1
  memmap/2 -> S_AXI_AWREADY/1
  memmap/1 -> S_AXI_ARREADY/1
  S_AXI_WVALID/1 -> memmap/25
  S_AXI_WSTRB/1 -> memmap/24
  S_AXI_WLAST/1 -> memmap/23
  S_AXI_WDATA/1 -> memmap/22
  S_AXI_RREADY/1 -> memmap/21
  S_AXI_BREADY/1 -> memmap/20
  S_AXI_AWVALID/1 -> memmap/19
  S_AXI_AWSIZE/1 -> memmap/18
  S_AXI_AWPROT/1 -> memmap/17
  S_AXI_AWLOCK/1 -> memmap/16
  S_AXI_AWLEN/1 -> memmap/15
  S_AXI_AWID/1 -> memmap/14
  S_AXI_AWCACHE/1 -> memmap/13
  S_AXI_AWBURST/1 -> memmap/12
  S_AXI_AWADDR/1 -> memmap/11
  S_AXI_ARVALID/1 -> memmap/10
  S_AXI_ARSIZE/1 -> memmap/9
  S_AXI_ARPROT/1 -> memmap/8
  S_AXI_ARLOCK/1 -> memmap/7
  S_AXI_ARLEN/1 -> memmap/6
  S_AXI_ARID/1 -> memmap/5
  S_AXI_ARCACHE/1 -> memmap/4
  S_AXI_ARBURST/1 -> memmap/3
  S_AXI_ARADDR/1 -> memmap/2
  AXI_ARESETN/1 -> memmap/1
  From Register4/1 -> memmap/30
  From Register/1 -> memmap/26
  From Register2/1 -> memmap/28
  From Register1/1 -> memmap/27
  To Register1/1 -> memmap/32
  To Register4/1 -> memmap/35
  To Register15/1 -> memmap/46
  To Register10/1 -> memmap/41
  To Register5/1 -> memmap/36
  To Register13/1 -> memmap/44
  To Register12/1 -> memmap/43
  To Register9/1 -> memmap/40
  To Register11/1 -> memmap/42
  To Register14/1 -> memmap/45
  To Register6/1 -> memmap/37
  To Register3/1 -> memmap/34
  To Register/1 -> memmap/31
  To Register7/1 -> memmap/38
  To Register2/1 -> memmap/33
  To Register8/1 -> memmap/39
  From Register3/1 -> memmap/29
  S_AXI_WREADY/1 -> Terminator10/1
  S_AXI_RVALID/1 -> Terminator9/1
  S_AXI_RRESP/1 -> Terminator8/1
  S_AXI_RLAST/1 -> Terminator7/1
  S_AXI_RID/1 -> Terminator6/1
  S_AXI_RDATA/1 -> Terminator5/1
  S_AXI_BVALID/1 -> Terminator4/1
  S_AXI_BRESP/1 -> Terminator3/1
  S_AXI_BID/1 -> Terminator2/1
  S_AXI_AWREADY/1 -> Terminator1/1
  S_AXI_ARREADY/1 -> Terminator/1
  Constant24/1 -> S_AXI_WVALID/1
  Constant23/1 -> S_AXI_WSTRB/1
  Constant22/1 -> S_AXI_WLAST/1
  Constant21/1 -> S_AXI_WDATA/1
  Constant20/1 -> S_AXI_RREADY/1
  Constant19/1 -> S_AXI_BREADY/1
  Constant18/1 -> S_AXI_AWVALID/1
  Constant17/1 -> S_AXI_AWSIZE/1
  Constant16/1 -> S_AXI_AWPROT/1
  Constant15/1 -> S_AXI_AWLOCK/1
  Constant14/1 -> S_AXI_AWLEN/1
  Constant13/1 -> S_AXI_AWID/1
  Constant12/1 -> S_AXI_AWCACHE/1
  Constant11/1 -> S_AXI_AWBURST/1
  Constant10/1 -> S_AXI_AWADDR/1
  Constant9/1 -> S_AXI_ARVALID/1
  Constant8/1 -> S_AXI_ARSIZE/1
  Constant7/1 -> S_AXI_ARPROT/1
  Constant6/1 -> S_AXI_ARLOCK/1
  Constant5/1 -> S_AXI_ARLEN/1
  Constant4/1 -> S_AXI_ARID/1
  Constant3/1 -> S_AXI_ARCACHE/1
  Constant2/1 -> S_AXI_ARBURST/1
  Constant1/1 -> S_AXI_ARADDR/1
  Constant/1 -> AXI_ARESETN/1
